simulink model "TosAeb"  (R2021a)
settings: Solver=FixedStepDiscrete  StartTime=0.0  StopTime=inf  FixedStep=0.01  RelTol=1e-3
undefined symbols (not defined in the file or in its folder): ACCModSts, AccSts1, AEB_ObjectMovingOrientation, AEB_ObjectMovingState, CN_BTCActive, CN_CurrentGearR, CN_CurrentGearR_DHT, CN_PTCActive, CN_VDCActive, CN_VSC_EspOff, CN_VSC_FCW_AEB_Error, CN_VSC_FCW_AEB_NoDispay, CN_VSC_FCW_AEB_PerfmDgdtn, CN_VSC_FCW_AEB_TempUnAvlbl, CN_VSC_VehStandstill, CNaccelAvoidMax, CNaccelAvoidMin, CNbrakePedalPress, CNcomfortStrLeftAccelMax, CNcomfortStrLeftAccelMin, CNcomfortStrRightAccelMax, CNcomfortStrRightAccelMin, CNDrvDoorOpen, CNDrvModeEco, CNDrvModeNorm, CNDrvModeOther, CNDrvModeSport, CNDrvSBRreminder, CNNull, CNobjInvalidID, CNPassDoorOpen, CNPassSBRreminder, CNPEBSAccelNegInf, CNPEBSAccelNull, CNPEBSTimeInf, CNPEBSTimeNull, CNSTOPMAX, CNSTOPNULL, CNsubjWidth, CNSwtOFF, CNSwtON, CNTTCMAX, CNTTCNULL, CNVehConfigAEBCycNone, CNVehConfigAEBNone, CNVehConfigAEBPedNone, CNVehConfigFCWNone, CNVehConfigJANone, CollisionSeverity, FALSE, FCWSnvty, JACycObjCalcInfoDefaultParams, JAPedObjCalcInfoDefaultParams, JAVehObjCalcInfoDefaultParams, latCycObjCalcInfoDefaultParams, latPedObjCalcInfoDefaultParams, longObjCalcInfoDefaultParams, ModSts, movingStatus, ObjectType ... (+1112)
libraries: simulink x3031, AEBSFunctionLib x50, Ta_Library x33, AEBSStateMachineLib x23, Mylibrary x12, simulink_extras x7, ObjSelect_lib x3, dspstat3 x2
(part 43 of 102)

== system TosAeb/FcwAebJa_Step_sys/ALG_AEBSystem/ALG_AEBController/AEB_FuncCondCalcApp/PedFunctionCondCalc/pedABASuppAborCond/pedABASuppCondCalc/pedABAObjRelActiveCondCalc ==
  ObjectID : Inport
  vxAbsObj : Inport  Port=2
  ObjFusQuality : Inport  Port=3
  dy : Inport  Port=4
  vy : Inport  Port=5
  Abs : Abs
  Compare To Constant : simulink/Logic and Bit Operations/Compare To Constant  relop=>  const=CNNull  OutDataTypeStr=boolean
  Compare To Constant1 : simulink/Logic and Bit Operations/Compare To Constant  relop=>  const=P_AEB_Func_pedABAVxAbsObjSuppThres  OutDataTypeStr=boolean
  Compare To Constant11 : simulink/Logic and Bit Operations/Compare To Constant  relop===  const=FALSE  OutDataTypeStr=boolean
  Compare To Constant2 : simulink/Logic and Bit Operations/Compare To Constant  relop=>  const=CNNull  OutDataTypeStr=boolean
  Compare To Constant3 : simulink/Logic and Bit Operations/Compare To Constant  relop=<  const=CNNull  OutDataTypeStr=boolean
  Compare To Constant4 : simulink/Logic and Bit Operations/Compare To Constant  relop===  const=CNobjInvalidID  OutDataTypeStr=boolean
  Compare To Constant5 : simulink/Logic and Bit Operations/Compare To Constant  relop=<  const=CNNull  OutDataTypeStr=boolean
  Logical Operator : Logic  Operator=OR  Inputs=3  AllPortsSameDT=off  OutDataTypeStr=boolean
  Logical Operator1 : Logic  AllPortsSameDT=off  OutDataTypeStr=boolean
  Logical Operator2 : Logic  AllPortsSameDT=off  OutDataTypeStr=boolean
  Logical Operator3 : Logic  Operator=OR  AllPortsSameDT=off  OutDataTypeStr=boolean
  Terminator : Terminator
  pedABAObjRelSuppCondFulfilled : Outport
  wires:
  ObjFusQuality/1 -> Compare To Constant11/1
  vy/1 -> Compare To Constant2/1, Compare To Constant5/1
  Compare To Constant2/1 -> Logical Operator1/2
  dy/1 -> Compare To Constant/1, Compare To Constant3/1
  Logical Operator2/1 -> Logical Operator3/2
  Compare To Constant/1 -> Logical Operator1/1
  Compare To Constant3/1 -> Logical Operator2/1
  Logical Operator1/1 -> Logical Operator3/1
  Compare To Constant5/1 -> Logical Operator2/2
  Compare To Constant11/1 -> Logical Operator/3  "ObjFusQuality"
  Logical Operator/1 -> pedABAObjRelSuppCondFulfilled/1
  Compare To Constant4/1 -> Logical Operator/1
  Compare To Constant1/1 -> Logical Operator/2
  vxAbsObj/1 -> Abs/1
  Abs/1 -> Compare To Constant1/1
  ObjectID/1 -> Compare To Constant4/1
  Logical Operator3/1 -> Terminator/1

== system TosAeb/FcwAebJa_Step_sys/ALG_AEBSystem/ALG_AEBController/AEB_FuncCondCalcApp/PedFunctionCondCalc/pedABASuppAborCond/pedABASuppCondCalc/pedABASituationRelActiveCondCalc ==
  latCollisionSeverity : Inport
  subjCurvTraj : Inport  Port=2
  subjLatAccel : Inport  Port=3
  ObjectTypeCameraConfirmed : Inport  Port=4
  vy : Inport  Port=5
  dxFrontBumper : Inport  Port=6
  colliisonRiskProb : Inport  Port=7
  FcwHMISetting : Inport  Port=8
  subjVel : Inport  Port=9
  LongDistCond4PreWarningABA : Subsystem
  pedABASituationRelSuppCondCalc : Subsystem
  vyCorrCalc : Subsystem
  pedABASituationRelSuppCondFulfilled : Outport
  wires:
  LongDistCond4PreWarningABA/1(longDistCond4ABA) -> pedABASituationRelSuppCondCalc/7(objLongDistCond4ABA)
  vyCorrCalc/1(objLatVyCond4ABA) -> pedABASituationRelSuppCondCalc/6(pedLatVyCond4ABA)
  pedABASituationRelSuppCondCalc/1(pedABASituationRelSuppCondFulfilled) -> pedABASituationRelSuppCondFulfilled/1
  colliisonRiskProb/1 -> pedABASituationRelSuppCondCalc/4(colliisonRiskProb)
  subjLatAccel/1 -> pedABASituationRelSuppCondCalc/3(subjLatAccel)
  subjCurvTraj/1 -> pedABASituationRelSuppCondCalc/2(subjCurvTraj)
  latCollisionSeverity/1 -> pedABASituationRelSuppCondCalc/1(latCollisionSeverity)
  subjVel/1 -> LongDistCond4PreWarningABA/2(subjVel)
  FcwHMISetting/1 -> LongDistCond4PreWarningABA/1(FcwHMISetting)
  dxFrontBumper/1 -> LongDistCond4PreWarningABA/3(dxFrontBumper)  "dxFrontBumper"
  ObjectTypeCameraConfirmed/1 -> pedABASituationRelSuppCondCalc/5(ObjectTypeCameraConfirmed), vyCorrCalc/1(ObjectTypeCameraConfirmed)
  vy/1 -> vyCorrCalc/2(vy)

== system TosAeb/FcwAebJa_Step_sys/ALG_AEBSystem/ALG_AEBController/AEB_FuncCondCalcApp/PedFunctionCondCalc/pedABASuppAborCond/pedABASuppCondCalc/pedABASuppCondFinalJudge ==
  pedABAObjRelSuppCondFulfilled : Inport
  pedABAVehRelSuppCondFulfilled : Inport  Port=2
  pedABASituationRelSuppCondFulfilled : Inport  Port=3
  Compare To Constant : simulink/Logic and Bit Operations/Compare To Constant  relop===  const=TRUE  OutDataTypeStr=boolean
  Compare To Constant1 : simulink/Logic and Bit Operations/Compare To Constant  relop===  const=TRUE  OutDataTypeStr=boolean
  Compare To Constant2 : simulink/Logic and Bit Operations/Compare To Constant  relop===  const=TRUE  OutDataTypeStr=boolean
  Logical Operator : Logic  Operator=OR  Inputs=3  AllPortsSameDT=off  OutDataTypeStr=boolean
  pedABASuppCondFulfilled : Outport
  wires:
  Logical Operator/1 -> pedABASuppCondFulfilled/1
  pedABASituationRelSuppCondFulfilled/1 -> Compare To Constant2/1
  pedABAObjRelSuppCondFulfilled/1 -> Compare To Constant/1
  pedABAVehRelSuppCondFulfilled/1 -> Compare To Constant1/1
  Compare To Constant/1 -> Logical Operator/1
  Compare To Constant1/1 -> Logical Operator/2
  Compare To Constant2/1 -> Logical Operator/3

== system TosAeb/FcwAebJa_Step_sys/ALG_AEBSystem/ALG_AEBController/AEB_FuncCondCalcApp/PedFunctionCondCalc/pedABASuppAborCond/pedABASuppCondCalc/pedABAVehRelActiveCondCalc ==
  subjVel : Inport
  SteerWheelAngle : Inport  Port=2
  SteerWheelSpd : Inport  Port=3
  AccrPedlPosnDiagc : Inport  Port=4
  vehStateCalcPedABASuppFlg : Inport  Port=5
  Abs : Abs
  Abs1 : Abs
  AccReleventSuppCondDelayAbortion : AEBSFunctionLib/StateDelayAbortion  SystemSampleTime=-1  VariantActivationTime=update diagram  Latency=0  AutoFrameSizeCalculation=off
  Compare To Constant : simulink/Logic and Bit Operations/Compare To Constant  relop=>  const=P_AEB_Func_pedABAStrWheelAngleSupThres  OutDataTypeStr=boolean
  Compare To Constant1 : simulink/Logic and Bit Operations/Compare To Constant  relop=>  const=P_AEB_Func_pedABAGasPedalSupThres  OutDataTypeStr=boolean
  Compare To Constant2 : simulink/Logic and Bit Operations/Compare To Constant  relop=>  const=P_AEB_Func_pedABAStrWheelAngleSpdSupThres  OutDataTypeStr=boolean
  Compare To Constant7 : simulink/Logic and Bit Operations/Compare To Constant  relop===  const=TRUE  OutDataTypeStr=boolean
  Compare To Constant8 : simulink/Logic and Bit Operations/Compare To Constant  relop=>  const=P_AEB_Func_pedABASubjVelMax  OutDataTypeStr=boolean
  Compare To Constant9 : simulink/Logic and Bit Operations/Compare To Constant  relop=<  const=P_AEB_Func_pedABASubjVelMin  OutDataTypeStr=boolean
  Constant : Constant  Value=P_AEB_Func_pedABAStrCondAborDelayTime
  Constant1 : Constant  Value=0.02
  Constant2 : Constant  Value=P_AEB_Func_pedABAAccrCondAborDelayTime
  Constant3 : Constant  Value=0.02
  Logical Operator : Logic  Operator=OR  AllPortsSameDT=off  OutDataTypeStr=boolean
  Logical Operator4 : Logic  Operator=OR  AllPortsSameDT=off  OutDataTypeStr=boolean
  Logical Operator5 : Logic  Operator=OR  Inputs=4  AllPortsSameDT=off  OutDataTypeStr=boolean
  StrReleventSuppCondDelayAbortion : AEBSFunctionLib/StateDelayAbortion  SystemSampleTime=-1  VariantActivationTime=update diagram  Latency=0  AutoFrameSizeCalculation=off
  pedABAVehRelSuppCondFulfilled : Outport
  wires:
  Compare To Constant1/1 -> AccReleventSuppCondDelayAbortion/1
  Constant2/1 -> AccReleventSuppCondDelayAbortion/2
  Constant3/1 -> AccReleventSuppCondDelayAbortion/3
  AccrPedlPosnDiagc/1 -> Compare To Constant1/1
  Logical Operator4/1 -> StrReleventSuppCondDelayAbortion/1
  Constant1/1 -> StrReleventSuppCondDelayAbortion/3
  Constant/1 -> StrReleventSuppCondDelayAbortion/2
  Logical Operator5/1 -> pedABAVehRelSuppCondFulfilled/1  "pedPrefillVehRelSuppCondFulfilled"
  subjVel/1 -> Compare To Constant9/1, Compare To Constant8/1
  Logical Operator/1 -> Logical Operator5/1  "subjVelReleventSuppCondFulfilled"
  Compare To Constant7/1 -> Logical Operator5/4  "vehStateCalcPedPrefillSuppCondFulfilled"
  Compare To Constant9/1 -> Logical Operator/2
  StrReleventSuppCondDelayAbortion/1 -> Logical Operator5/2  "strReleventSuppCondFulfilled"
  SteerWheelAngle/1 -> Abs/1
  Abs/1 -> Compare To Constant/1
  Compare To Constant/1 -> Logical Operator4/1
  SteerWheelSpd/1 -> Abs1/1
  Compare To Constant2/1 -> Logical Operator4/2
  Compare To Constant8/1 -> Logical Operator/1
  vehStateCalcPedABASuppFlg/1 -> Compare To Constant7/1
  Abs1/1 -> Compare To Constant2/1
  AccReleventSuppCondDelayAbortion/1 -> Logical Operator5/3  "accReleventSuppCondFulfilled"

== system TosAeb/FcwAebJa_Step_sys/ALG_AEBSystem/ALG_AEBController/AEB_FuncCondCalcApp/PedFunctionCondCalc/pedAEBfullSuppAborCond/pedAEBfullAborCondNoStandstillCalc/pedAEBfullAborCondFinalJudge ==
  pedAEBfullObjRelAborCondFulfilled : Inport
  pedAEBfullVehRelAborCondFulfilled : Inport  Port=2
  pedAEBfullSituationRelAborCondFulfilled : Inport  Port=3
  Compare To Constant1 : simulink/Logic and Bit Operations/Compare To Constant  relop===  const=TRUE  OutDataTypeStr=boolean
  Compare To Constant2 : simulink/Logic and Bit Operations/Compare To Constant  relop===  const=TRUE  OutDataTypeStr=boolean
  Compare To Constant3 : simulink/Logic and Bit Operations/Compare To Constant  relop===  const=TRUE  OutDataTypeStr=boolean
  Logical Operator : Logic  Operator=OR  Inputs=3  AllPortsSameDT=off  OutDataTypeStr=boolean
  pedAEBfullAborCondNoStandstillFulfilled : Outport
  wires:
  Compare To Constant2/1 -> Logical Operator/3
  Compare To Constant1/1 -> Logical Operator/2
  pedAEBfullObjRelAborCondFulfilled/1 -> Compare To Constant3/1
  Logical Operator/1 -> pedAEBfullAborCondNoStandstillFulfilled/1  "pedAEBfullAborCondNoStandstillFulfilled"
  pedAEBfullSituationRelAborCondFulfilled/1 -> Compare To Constant2/1
  pedAEBfullVehRelAborCondFulfilled/1 -> Compare To Constant1/1
  Compare To Constant3/1 -> Logical Operator/1

== system TosAeb/FcwAebJa_Step_sys/ALG_AEBSystem/ALG_AEBController/AEB_FuncCondCalcApp/PedFunctionCondCalc/pedAEBfullSuppAborCond/pedAEBfullAborCondNoStandstillCalc/pedAEBfullObjRelActiveCondCalc ==
  ObjectID : Inport
  objIsFusionTarget : Inport  Port=2
  latCollsionRiskProb : Inport  Port=3
  Chart : Stateflow chart  TreatAsAtomicUnit=on
  Compare To Constant : simulink/Logic and Bit Operations/Compare To Constant  relop===  const=TRUE  OutDataTypeStr=boolean
  Constant : Constant  Value=P_AEB_Func_pedAEBfulColliisonRiskProbAborThresObjID
  Unit Delay : UnitDelay  SampleTime=-1  HasFrameUpgradeWarning=on
  pedAEBfullObjRelAborCondFulfilled : Outport
  wires:
  Constant/1 -> Chart/5(P_pedAEBfulColliisonRiskProbAborThresObjID)
  Compare To Constant/1 -> Chart/3(objIsFusionTarget)
  objIsFusionTarget/1 -> Compare To Constant/1
  ObjectID/1 -> Chart/1(targetObjID), Unit Delay/1
  Chart/1(pedAEBfullObjRelAborCondFulfilled) -> pedAEBfullObjRelAborCondFulfilled/1
  Unit Delay/1 -> Chart/2(targetObjIDObjCalcK1)
  latCollsionRiskProb/1 -> Chart/4(objLatCollisionProb)

== system TosAeb/FcwAebJa_Step_sys/ALG_AEBSystem/ALG_AEBController/AEB_FuncCondCalcApp/PedFunctionCondCalc/pedAEBfullSuppAborCond/pedAEBfullAborCondNoStandstillCalc/pedAEBfullSituationRelAborCondCalc ==
  pedVehVelReduced : Inport
  pedCollisionSeverity : Inport  Port=2
  objLatCollsionRiskProb : Inport  Port=3
  dy : Inport  Port=4
  vy : Inport  Port=5
  dx : Inport  Port=6
  subjYawRate : Inport  Port=7
  Abs : Abs
  Abs1 : Abs
  Add : Sum
  Compare To Constant : simulink/Logic and Bit Operations/Compare To Constant  relop===  const=CollisionSeverity.None  OutDataTypeStr=boolean
  Compare To Constant1 : simulink/Logic and Bit Operations/Compare To Constant  relop=>  const=P_AEB_Func_pedAEBfullVehVelReduceAborThres  OutDataTypeStr=boolean
  Compare To Constant2 : simulink/Logic and Bit Operations/Compare To Constant  relop=>  const=P_AEB_Func_pedAEBfullAbsDyAborThres  OutDataTypeStr=boolean
  Compare To Constant3 : simulink/Logic and Bit Operations/Compare To Constant  relop=<  const=P_AEB_Func_pedAEBfullColliisonRiskProbAborThres  OutDataTypeStr=boolean
  Compare To Constant4 : simulink/Logic and Bit Operations/Compare To Constant  relop=<  const=P_AEB_Func_pedAEBfullAbsVyAborThres  OutDataTypeStr=boolean
  Logical Operator : Logic  AllPortsSameDT=off  OutDataTypeStr=boolean
  Logical Operator1 : Logic  Operator=OR  Inputs=4  AllPortsSameDT=off  OutDataTypeStr=boolean
  Product : Product
  pedAEBfullSituationRelAborCondFulfilled : Outport
  wires:
  Compare To Constant1/1 -> Logical Operator1/1
  Compare To Constant/1 -> Logical Operator1/2
  Add/1 -> Abs1/1  "vyCorr"
  dx/1 -> Product/1
  subjYawRate/1 -> Product/2
  vy/1 -> Add/1
  Product/1 -> Add/2
  Logical Operator1/1 -> pedAEBfullSituationRelAborCondFulfilled/1
  Compare To Constant3/1 -> Logical Operator1/3
  pedCollisionSeverity/1 -> Compare To Constant/1
  objLatCollsionRiskProb/1 -> Compare To Constant3/1
  pedVehVelReduced/1 -> Compare To Constant1/1
  dy/1 -> Abs/1
  Abs/1 -> Compare To Constant2/1
  Abs1/1 -> Compare To Constant4/1
  Compare To Constant2/1 -> Logical Operator/1
  Compare To Constant4/1 -> Logical Operator/2
  Logical Operator/1 -> Logical Operator1/4

== system TosAeb/FcwAebJa_Step_sys/ALG_AEBSystem/ALG_AEBController/AEB_FuncCondCalcApp/PedFunctionCondCalc/pedAEBfullSuppAborCond/pedAEBfullAborCondNoStandstillCalc/pedAEBfullVehRelAborCondCalc ==
  note: 取消自车速度判断条件
  SteerWheelAngle : Inport
  SteerWheelSpd : Inport  Port=2
  vehStateCalcAEBfullAborFlg : Inport  Port=3
  pedAEBfullWorkTime : Inport  Port=4
  vehStateCalcStandstill : Inport  Port=5
  Abs : Abs
  Abs1 : Abs
  Compare To Constant : simulink/Logic and Bit Operations/Compare To Constant  relop=>  const=P_AEB_Func_pedAEBfullStrWheelAngleAborThres  OutDataTypeStr=boolean
  Compare To Constant1 : simulink/Logic and Bit Operations/Compare To Constant  relop=>  const=P_AEB_Func_pedAEBfullStrWheelAngleSpdAborThres  OutDataTypeStr=boolean
  Compare To Constant2 : simulink/Logic and Bit Operations/Compare To Constant  relop=>  const=P_AEB_Func_pedAEBfullActivationTimeAborThres  OutDataTypeStr=boolean
  Compare To Constant3 : simulink/Logic and Bit Operations/Compare To Constant  relop===  const=TRUE  OutDataTypeStr=boolean
  Compare To Constant4 : simulink/Logic and Bit Operations/Compare To Constant  relop===  const=FALSE  OutDataTypeStr=boolean
  Logical Operator : Logic  Operator=OR  AllPortsSameDT=off  OutDataTypeStr=boolean
  Logical Operator2 : Logic  Operator=OR  Inputs=3  AllPortsSameDT=off  OutDataTypeStr=boolean
  Logical Operator4 : Logic  AllPortsSameDT=off  OutDataTypeStr=boolean
  pedAEBfullVehRelAborCondFulfilled : Outport
  wires:
  Compare To Constant3/1 -> Logical Operator2/2  "vehStateCalcAEBfullAborFlg"
  Logical Operator4/1 -> Logical Operator2/3  "pedPrefillWorkTimeRelCondFulfilled"
  Abs/1 -> Compare To Constant/1
  SteerWheelSpd/1 -> Abs1/1
  Abs1/1 -> Compare To Constant1/1
  Compare To Constant1/1 -> Logical Operator/2
  SteerWheelAngle/1 -> Abs/1
  pedAEBfullWorkTime/1 -> Compare To Constant2/1
  vehStateCalcAEBfullAborFlg/1 -> Compare To Constant3/1
  Compare To Constant/1 -> Logical Operator/1
  Logical Operator/1 -> Logical Operator2/1  "strReleventAborCondFulfilled"
  Logical Operator2/1 -> pedAEBfullVehRelAborCondFulfilled/1  "pedPrefillVehRelAborCondFulfilled"
  vehStateCalcStandstill/1 -> Compare To Constant4/1
  Compare To Constant4/1 -> Logical Operator4/1
  Compare To Constant2/1 -> Logical Operator4/2

== system TosAeb/FcwAebJa_Step_sys/ALG_AEBSystem/ALG_AEBController/AEB_FuncCondCalcApp/PedFunctionCondCalc/pedAEBfullSuppAborCond/pedAEBfullAborCondStandstillCalc/pedAEBfullVehRelAborCondCalc ==
  note: 取消速度限制
  subjVel : Inport
  SteerWheelAngle : Inport  Port=2
  SteerWheelSpd : Inport  Port=3
  vehStateCalcAEBfullAborFlg : Inport  Port=4
  pedAEBfullWorkTime : Inport  Port=5
  vehStateCalcStandstill : Inport  Port=6
  Abs : Abs
  Abs1 : Abs
  Compare To Constant : simulink/Logic and Bit Operations/Compare To Constant  relop=>  const=P_AEB_Func_pedAEBfullStrWheelAngleAborThresStandstill  OutDataTypeStr=boolean
  Compare To Constant1 : simulink/Logic and Bit Operations/Compare To Constant  relop=>  const=P_AEB_Func_pedAEBfullStrWheelAngleSpdAborThresStandstill  OutDataTypeStr=boolean
  Compare To Constant2 : simulink/Logic and Bit Operations/Compare To Constant  relop=>  const=P_AEB_Func_pedAEBfullActivationTimeAborThresStandstill  OutDataTypeStr=boolean
  Compare To Constant3 : simulink/Logic and Bit Operations/Compare To Constant  relop===  const=TRUE  OutDataTypeStr=boolean
  Compare To Constant4 : simulink/Logic and Bit Operations/Compare To Constant  relop===  const=TRUE  OutDataTypeStr=boolean
  Compare To Constant7 : simulink/Logic and Bit Operations/Compare To Constant  relop=<  const=P_AEB_Func_pedAEBfullSubjVelAborThresStandstill  OutDataTypeStr=boolean
  Logical Operator : Logic  Operator=OR  AllPortsSameDT=off  OutDataTypeStr=boolean
  Logical Operator2 : Logic  Operator=OR  Inputs=3  AllPortsSameDT=off  OutDataTypeStr=boolean
  Logical Operator4 : Logic  Inputs=3  AllPortsSameDT=off  OutDataTypeStr=boolean
  pedAEBfullVehRelAborCondFulfilled : Outport
  wires:
  Logical Operator4/1 -> Logical Operator2/3  "pedPrefillWorkTimeRelCondFulfilled"
  vehStateCalcStandstill/1 -> Compare To Constant4/1
  subjVel/1 -> Compare To Constant7/1
  Logical Operator2/1 -> pedAEBfullVehRelAborCondFulfilled/1  "pedPrefillVehRelAborCondFulfilled"
  Logical Operator/1 -> Logical Operator2/1  "strReleventAborCondFulfilled"
  Compare To Constant/1 -> Logical Operator/1
  vehStateCalcAEBfullAborFlg/1 -> Compare To Constant3/1
  pedAEBfullWorkTime/1 -> Compare To Constant2/1
  SteerWheelAngle/1 -> Abs/1
  Compare To Constant1/1 -> Logical Operator/2
  Abs1/1 -> Compare To Constant1/1
  SteerWheelSpd/1 -> Abs1/1
  Abs/1 -> Compare To Constant/1
  Compare To Constant3/1 -> Logical Operator2/2  "vehStateCalcAEBfullAborFlg"
  Compare To Constant4/1 -> Logical Operator4/2
  Compare To Constant7/1 -> Logical Operator4/1
  Compare To Constant2/1 -> Logical Operator4/3

== system TosAeb/FcwAebJa_Step_sys/ALG_AEBSystem/ALG_AEBController/AEB_FuncCondCalcApp/PedFunctionCondCalc/pedAEBfullSuppAborCond/pedAEBfullSuppCondCalc/pedAEBfullObjRelSuppCondCalc ==
  ObjectID : Inport
  vxAbsObj : Inport  Port=2
  ObjFusQuality : Inport  Port=3
  dy : Inport  Port=4
  vy : Inport  Port=5
  Abs : Abs
  Compare To Constant : simulink/Logic and Bit Operations/Compare To Constant  relop=>  const=CNNull  OutDataTypeStr=boolean
  Compare To Constant1 : simulink/Logic and Bit Operations/Compare To Constant  relop=>  const=P_AEB_Func_pedAEBfullVxAbsObjSuppThres  OutDataTypeStr=boolean
  Compare To Constant11 : simulink/Logic and Bit Operations/Compare To Constant  relop===  const=FALSE  OutDataTypeStr=boolean
  Compare To Constant2 : simulink/Logic and Bit Operations/Compare To Constant  relop=>  const=CNNull  OutDataTypeStr=boolean
  Compare To Constant3 : simulink/Logic and Bit Operations/Compare To Constant  relop=<  const=CNNull  OutDataTypeStr=boolean
  Compare To Constant4 : simulink/Logic and Bit Operations/Compare To Constant  relop===  const=CNobjInvalidID  OutDataTypeStr=boolean
  Compare To Constant5 : simulink/Logic and Bit Operations/Compare To Constant  relop=<  const=CNNull  OutDataTypeStr=boolean
  Logical Operator : Logic  Operator=OR  Inputs=3  AllPortsSameDT=off  OutDataTypeStr=boolean
  Logical Operator1 : Logic  AllPortsSameDT=off  OutDataTypeStr=boolean
  Logical Operator2 : Logic  AllPortsSameDT=off  OutDataTypeStr=boolean
  Logical Operator3 : Logic  Operator=OR  AllPortsSameDT=off  OutDataTypeStr=boolean
  Terminator : Terminator
  pedAEBfullObjRelSuppCondFulfilled : Outport
  wires:
  ObjFusQuality/1 -> Compare To Constant11/1
  vy/1 -> Compare To Constant2/1, Compare To Constant5/1
  Compare To Constant2/1 -> Logical Operator1/2
  dy/1 -> Compare To Constant/1, Compare To Constant3/1
  Logical Operator2/1 -> Logical Operator3/2
  Compare To Constant/1 -> Logical Operator1/1
  Compare To Constant3/1 -> Logical Operator2/1
  Logical Operator1/1 -> Logical Operator3/1
  Compare To Constant5/1 -> Logical Operator2/2
  Compare To Constant11/1 -> Logical Operator/3  "ObjFusQuality"
  vxAbsObj/1 -> Abs/1
  Compare To Constant1/1 -> Logical Operator/2
  Abs/1 -> Compare To Constant1/1
  Compare To Constant4/1 -> Logical Operator/1
  Logical Operator/1 -> pedAEBfullObjRelSuppCondFulfilled/1
  ObjectID/1 -> Compare To Constant4/1
  Logical Operator3/1 -> Terminator/1

== system TosAeb/FcwAebJa_Step_sys/ALG_AEBSystem/ALG_AEBController/AEB_FuncCondCalcApp/PedFunctionCondCalc/pedAEBfullSuppAborCond/pedAEBfullSuppCondCalc/pedAEBfullSituationRelSuppCondCalc ==
  latCollisionSeverity : Inport
  subjCurvTraj : Inport  Port=2
  subjLatAccel : Inport  Port=3
  pedCollisionProbFlg : Inport  Port=4
  pedCollisionRisk : Inport  Port=5
  dy : Inport  Port=6
  FcwHMISetting : Inport  Port=7
  subjVel : Inport  Port=8
  dxFrontBumper : Inport  Port=9
  ObjectTypeCameraConfirmed : Inport  Port=10
  distSecure : Inport  Port=11
  distBraking : Inport  Port=12
  vy : Inport  Port=13
  dy_ConfirmCalc : Subsystem  Commented=on
  latDistCondCalc : Subsystem
  longDistCond4fullBrkCalc : Subsystem
  objLatVyCond : Subsystem
  pedAEBfullSituationRelSuppCond : Subsystem
  vy_ConfirmCalc : Subsystem  Commented=on
  pedAEBfullSituationRelSuppCondFulfilled : Outport
  wires:
  [dy] -> dy_ConfirmCalc/3(dy)
  [subjVel] -> dy_ConfirmCalc/1(subjVel)
  [dy] -> vy_ConfirmCalc/2(dy)
  dxFrontBumper/1 -> longDistCond4fullBrkCalc/1(dxFrontBumper)
  [subjVel] -> longDistCond4fullBrkCalc/4(subjVel)
  distBraking/1 -> longDistCond4fullBrkCalc/3(distBraking)
  distSecure/1 -> longDistCond4fullBrkCalc/2(distSecure)
  longDistCond4fullBrkCalc/2(longDistMinCond4Brake) -> pedAEBfullSituationRelSuppCond/10(distMinCond4FullBrake)
  longDistCond4fullBrkCalc/1(longDistCond4fullBrk) -> pedAEBfullSituationRelSuppCond/9(objLongDistCond4FullBrake)
  latDistCondCalc/1(latDistCond) -> pedAEBfullSituationRelSuppCond/8(latDistCond)
  objLatVyCond/1(objLatVyCond4FullBrake) -> pedAEBfullSituationRelSuppCond/7(objLatVyCond4FullBrake)
  ObjectTypeCameraConfirmed/1 -> objLatVyCond/1(ObjectTypeCameraConfirmed), pedAEBfullSituationRelSuppCond/6(ObjectTypeCameraConfirmed)
  pedAEBfullSituationRelSuppCond/1(pedAEBfullSituationRelSuppCondFulfilled) -> pedAEBfullSituationRelSuppCondFulfilled/1
  pedCollisionRisk/1 -> pedAEBfullSituationRelSuppCond/5(pedCollisionRisk)
  pedCollisionProbFlg/1 -> pedAEBfullSituationRelSuppCond/4(pedCollisionProbFlg)
  subjLatAccel/1 -> pedAEBfullSituationRelSuppCond/3(subjLatAccel)
  subjCurvTraj/1 -> pedAEBfullSituationRelSuppCond/2(subjCurvTraj)
  latCollisionSeverity/1 -> pedAEBfullSituationRelSuppCond/1(latCollisionSeverity)
  dy/1 -> objLatVyCond/3(dy), latDistCondCalc/1(dy), [dy]
  FcwHMISetting/1 -> latDistCondCalc/2(FcwHMISetting)
  subjVel/1 -> latDistCondCalc/3(subjVel), [subjVel]
  vy/1 -> latDistCondCalc/4(vy), vy_ConfirmCalc/1(vy), objLatVyCond/2(vy)

== system TosAeb/FcwAebJa_Step_sys/ALG_AEBSystem/ALG_AEBController/AEB_FuncCondCalcApp/PedFunctionCondCalc/pedAEBfullSuppAborCond/pedAEBfullSuppCondCalc/pedAEBfullSuppCondFinalJudge ==
  pedAEBfullObjRelSuppCondFulfilled : Inport
  pedAEBfullVehRelSuppCondFulfilled : Inport  Port=2
  pedAEBfullSituationRelSuppCondFulfilled : Inport  Port=3
  Compare To Constant : simulink/Logic and Bit Operations/Compare To Constant  relop===  const=TRUE  OutDataTypeStr=boolean
  Compare To Constant1 : simulink/Logic and Bit Operations/Compare To Constant  relop===  const=TRUE  OutDataTypeStr=boolean
  Compare To Constant2 : simulink/Logic and Bit Operations/Compare To Constant  relop===  const=TRUE  OutDataTypeStr=boolean
  Logical Operator : Logic  Operator=OR  Inputs=3  AllPortsSameDT=off  OutDataTypeStr=boolean
  pedAEBfullSuppCondFulfilled : Outport
  wires:
  Logical Operator/1 -> pedAEBfullSuppCondFulfilled/1
  pedAEBfullSituationRelSuppCondFulfilled/1 -> Compare To Constant2/1
  pedAEBfullObjRelSuppCondFulfilled/1 -> Compare To Constant/1
  pedAEBfullVehRelSuppCondFulfilled/1 -> Compare To Constant1/1
  Compare To Constant/1 -> Logical Operator/1
  Compare To Constant1/1 -> Logical Operator/2
  Compare To Constant2/1 -> Logical Operator/3

== system TosAeb/FcwAebJa_Step_sys/ALG_AEBSystem/ALG_AEBController/AEB_FuncCondCalcApp/PedFunctionCondCalc/pedAEBfullSuppAborCond/pedAEBfullSuppCondCalc/pedAEBfullVehRelSuppCondCalc ==
  subjVel : Inport
  VehConfigIbooster : Inport  Port=2
  ESP_MasterCylindBrakePressValid : Inport  Port=3
  ESP_MasterCylindBrakePress : Inport  Port=4
  SteerWheelAngle : Inport  Port=5
  SteerWheelSpd : Inport  Port=6
  AccrPedlPosnDiagc : Inport  Port=7
  vehStateCalcPedAEBfullSuppFlg : Inport  Port=8
  Abs : Abs
  Abs1 : Abs
  AccReleventSuppCondDelayAbortion : AEBSFunctionLib/StateDelayAbortion  SystemSampleTime=-1  VariantActivationTime=update diagram  Latency=0  AutoFrameSizeCalculation=off
  Compare To Constant : simulink/Logic and Bit Operations/Compare To Constant  relop=>  const=P_AEB_Func_pedAEBfullStrWheelAngleSupThres  OutDataTypeStr=boolean
  Compare To Constant1 : simulink/Logic and Bit Operations/Compare To Constant  relop=>  const=P_AEB_Func_pedAEBfullGasPedalSupThres  OutDataTypeStr=boolean
  Compare To Constant2 : simulink/Logic and Bit Operations/Compare To Constant  relop=>  const=P_AEB_Func_pedAEBfullStrWheelAngleSpdSupThres  OutDataTypeStr=boolean
  Compare To Constant7 : simulink/Logic and Bit Operations/Compare To Constant  relop===  const=TRUE  OutDataTypeStr=boolean
  Compare To Constant8 : simulink/Logic and Bit Operations/Compare To Constant  relop=>  const=P_AEB_Func_pedAEBfullSubjVelMax  OutDataTypeStr=boolean
  Compare To Constant9 : simulink/Logic and Bit Operations/Compare To Constant  relop=<  const=P_AEB_Func_pedAEBfullSubjVelMin  OutDataTypeStr=boolean
  Constant : Constant  Value=P_AEB_Func_pedAEBfullStrCondAborDelayTime
  Constant1 : Constant  Value=0.02
  Constant2 : Constant  Value=P_AEB_Func_pedAEBfullStrCondAborDelayTime
  Constant3 : Constant  Value=0.02
  Logical Operator : Logic  Operator=OR  AllPortsSameDT=off  OutDataTypeStr=boolean
  Logical Operator4 : Logic  Operator=OR  AllPortsSameDT=off  OutDataTypeStr=boolean
  Logical Operator5 : Logic  Operator=OR  Inputs=5  AllPortsSameDT=off  OutDataTypeStr=boolean
  StrReleventSuppCondDelayAbortion : AEBSFunctionLib/StateDelayAbortion  SystemSampleTime=-1  VariantActivationTime=update diagram  Latency=0  AutoFrameSizeCalculation=off
  brakeReleventSuppCond : Subsystem
  pedAEBfullVehRelSuppCondFulfilled : Outport
  wires:
  VehConfigIbooster/1 -> brakeReleventSuppCond/1(VehConfigIbooster)
  ESP_MasterCylindBrakePress/1 -> brakeReleventSuppCond/3(ESP_MasterCylindBrakePress)
  ESP_MasterCylindBrakePressValid/1 -> brakeReleventSuppCond/2(ESP_MasterCylindBrakePressValid)
  Compare To Constant1/1 -> AccReleventSuppCondDelayAbortion/1
  Constant2/1 -> AccReleventSuppCondDelayAbortion/2
  Constant3/1 -> AccReleventSuppCondDelayAbortion/3
  AccrPedlPosnDiagc/1 -> Compare To Constant1/1
  Logical Operator4/1 -> StrReleventSuppCondDelayAbortion/1
  Constant1/1 -> StrReleventSuppCondDelayAbortion/3
  Constant/1 -> StrReleventSuppCondDelayAbortion/2
  Logical Operator5/1 -> pedAEBfullVehRelSuppCondFulfilled/1  "pedAEBfullVehRelSuppCondFulfilled"
  subjVel/1 -> Compare To Constant9/1, Compare To Constant8/1
  Logical Operator/1 -> Logical Operator5/1  "subjVelReleventSuppCondFulfilled"
  Compare To Constant7/1 -> Logical Operator5/5  "vehStateCalcPedPrefillSuppCondFulfilled"
  Compare To Constant9/1 -> Logical Operator/2
  brakeReleventSuppCond/1(brakeReleventSuppCond) -> Logical Operator5/2  "BrkReleventSuppCondFulfilled"
  StrReleventSuppCondDelayAbortion/1 -> Logical Operator5/3  "strReleventSuppCondFulfilled"
  SteerWheelAngle/1 -> Abs/1
  Abs/1 -> Compare To Constant/1
  Compare To Constant/1 -> Logical Operator4/1
  SteerWheelSpd/1 -> Abs1/1
  Compare To Constant2/1 -> Logical Operator4/2
  Compare To Constant8/1 -> Logical Operator/1
  vehStateCalcPedAEBfullSuppFlg/1 -> Compare To Constant7/1
  Abs1/1 -> Compare To Constant2/1
  AccReleventSuppCondDelayAbortion/1 -> Logical Operator5/4  "accReleventSuppCondFulfilled"

== system TosAeb/FcwAebJa_Step_sys/ALG_AEBSystem/ALG_AEBController/AEB_FuncCondCalcApp/PedFunctionCondCalc/pedPreWarningSuppAborCond/pedPreWarningAborCondCalc/pedPreWarningAborCondFinalJudge ==
  pedPreWarningObjRelAborCondFulfilled : Inport
  pedPreWarningVehRelAborCondFulfilled : Inport  Port=2
  pedPreWarningSituationRelAborCondFulfilled : Inport  Port=3
  Compare To Constant1 : simulink/Logic and Bit Operations/Compare To Constant  relop===  const=TRUE  OutDataTypeStr=boolean
  Compare To Constant2 : simulink/Logic and Bit Operations/Compare To Constant  relop===  const=TRUE  OutDataTypeStr=boolean
  Compare To Constant3 : simulink/Logic and Bit Operations/Compare To Constant  relop===  const=TRUE  OutDataTypeStr=boolean
  Logical Operator : Logic  Operator=OR  Inputs=3  AllPortsSameDT=off  OutDataTypeStr=boolean
  pedPreWarningAborCondFulfilled : Outport
  wires:
  Compare To Constant3/1 -> Logical Operator/1
  pedPreWarningVehRelAborCondFulfilled/1 -> Compare To Constant1/1
  pedPreWarningSituationRelAborCondFulfilled/1 -> Compare To Constant2/1
  Logical Operator/1 -> pedPreWarningAborCondFulfilled/1  "vehPreWarningAborCondFulfilled"
  pedPreWarningObjRelAborCondFulfilled/1 -> Compare To Constant3/1
  Compare To Constant1/1 -> Logical Operator/2
  Compare To Constant2/1 -> Logical Operator/3

== system TosAeb/FcwAebJa_Step_sys/ALG_AEBSystem/ALG_AEBController/AEB_FuncCondCalcApp/PedFunctionCondCalc/pedPreWarningSuppAborCond/pedPreWarningAborCondCalc/pedPreWarningObjRelActiveCondCalc ==
  ObjectID : Inport
  pedPreWarningWorkTime : Inport  Port=2
  Compare To Constant : simulink/Logic and Bit Operations/Compare To Constant  relop=>=  const=P_AEB_Func_pedPrewarningMinActivationTimeAborThres  OutDataTypeStr=boolean
  Compare To Constant1 : simulink/Logic and Bit Operations/Compare To Constant  relop=>=  const=P_AEB_Func_pedPrewarningMinActivationTimeAborThresObjID  OutDataTypeStr=boolean
  Compare To Constant4 : simulink/Logic and Bit Operations/Compare To Constant  relop=~=  const=CNobjInvalidID  OutDataTypeStr=boolean
  Logical Operator : Logic  AllPortsSameDT=off  OutDataTypeStr=boolean
  Relational Operator : RelationalOperator  Operator=~=  OutDataTypeStr=boolean  RndMeth=Simplest
  Switch : Switch  Criteria=u2 ~= 0
  Unit Delay : UnitDelay  SampleTime=-1  HasFrameUpgradeWarning=on
  pedPreWarningObjRelAborCondFulfilled : Outport
  wires:
  Compare To Constant4/1 -> Switch/2
  pedPreWarningWorkTime/1 -> Compare To Constant/1, Compare To Constant1/1
  Compare To Constant/1 -> Switch/3
  ObjectID/1 -> Unit Delay/1, Relational Operator/2, Compare To Constant4/1
  Unit Delay/1 -> Relational Operator/1
  Compare To Constant1/1 -> Logical Operator/1
  Relational Operator/1 -> Logical Operator/2
  Switch/1 -> pedPreWarningObjRelAborCondFulfilled/1
  Logical Operator/1 -> Switch/1

== system TosAeb/FcwAebJa_Step_sys/ALG_AEBSystem/ALG_AEBController/AEB_FuncCondCalcApp/PedFunctionCondCalc/pedPreWarningSuppAborCond/pedPreWarningAborCondCalc/pedPreWarningSituationRelAborCondCalc ==
  note: 将true改为false
  latCollisionSeverity : Inport
  pedPreWarningWorkTime : Inport  Port=2
  Compare To Constant : simulink/Logic and Bit Operations/Compare To Constant  relop===  const=CollisionSeverity.None  OutDataTypeStr=boolean
  Compare To Constant1 : simulink/Logic and Bit Operations/Compare To Constant  relop=>=  const=P_AEB_Func_pedPrewarningMinActivationTimeAborThresSeverity  OutDataTypeStr=boolean
  Logical Operator : Logic  AllPortsSameDT=off  OutDataTypeStr=boolean
  pedPreWarningSituationRelAborCondFulfilled : Outport
  wires:
  Compare To Constant1/1 -> Logical Operator/2
  pedPreWarningWorkTime/1 -> Compare To Constant1/1
  Compare To Constant/1 -> Logical Operator/1
  latCollisionSeverity/1 -> Compare To Constant/1
  Logical Operator/1 -> pedPreWarningSituationRelAborCondFulfilled/1

== system TosAeb/FcwAebJa_Step_sys/ALG_AEBSystem/ALG_AEBController/AEB_FuncCondCalcApp/PedFunctionCondCalc/pedPreWarningSuppAborCond/pedPreWarningAborCondCalc/pedPreWarningVehRelAborCondCalc ==
  note: 取消速度限制
  vehStateCalcPreWarningAborFlg : Inport
  pedPreWarningWorkTime : Inport  Port=2
  Compare To Constant2 : simulink/Logic and Bit Operations/Compare To Constant  relop=>=  const=P_AEB_Func_pedPrewarningMaxActivationTimeAborThres  OutDataTypeStr=boolean
  Compare To Constant3 : simulink/Logic and Bit Operations/Compare To Constant  relop===  const=TRUE  OutDataTypeStr=boolean
  Compare To Constant4 : simulink/Logic and Bit Operations/Compare To Constant  relop=>=  const=P_AEB_Func_pedPrewarningMinActivationTimeAborThresVSC  OutDataTypeStr=boolean
  Logical Operator1 : Logic  AllPortsSameDT=off  OutDataTypeStr=boolean
  Logical Operator2 : Logic  Operator=OR  AllPortsSameDT=off  OutDataTypeStr=boolean
  pedPreWarningVehRelAborCondFulfilled : Outport
  wires:
  Logical Operator2/1 -> pedPreWarningVehRelAborCondFulfilled/1
  vehStateCalcPreWarningAborFlg/1 -> Compare To Constant3/1
  pedPreWarningWorkTime/1 -> Compare To Constant4/1, Compare To Constant2/1
  Compare To Constant2/1 -> Logical Operator2/2  "pedPreWarningWorkTimeRelCondFulfilled"
  Logical Operator1/1 -> Logical Operator2/1  "vehStateCalcPrefillAborCondFulfilled"
  Compare To Constant4/1 -> Logical Operator1/2
  Compare To Constant3/1 -> Logical Operator1/1

== system TosAeb/FcwAebJa_Step_sys/ALG_AEBSystem/ALG_AEBController/AEB_FuncCondCalcApp/PedFunctionCondCalc/pedPreWarningSuppAborCond/pedPreWarningSuppCondCalc/pedPreWarningObjRelActiveCondCalc ==
  ObjectID : Inport
  vxAbsObj : Inport  Port=2
  ObjFusQuality : Inport  Port=3
  dy : Inport  Port=4
  vy : Inport  Port=5
  Abs : Abs
  Compare To Constant : simulink/Logic and Bit Operations/Compare To Constant  relop=>  const=CNNull  OutDataTypeStr=boolean
  Compare To Constant1 : simulink/Logic and Bit Operations/Compare To Constant  relop=>  const=P_AEB_Func_pedPreWarningVxAbsObjSuppThres  OutDataTypeStr=boolean
  Compare To Constant2 : simulink/Logic and Bit Operations/Compare To Constant  relop===  const=FALSE  OutDataTypeStr=boolean
  Compare To Constant3 : simulink/Logic and Bit Operations/Compare To Constant  relop=>  const=CNNull  OutDataTypeStr=boolean
  Compare To Constant4 : simulink/Logic and Bit Operations/Compare To Constant  relop===  const=CNobjInvalidID  OutDataTypeStr=boolean
  Compare To Constant5 : simulink/Logic and Bit Operations/Compare To Constant  relop=<  const=CNNull  OutDataTypeStr=boolean
  Compare To Constant6 : simulink/Logic and Bit Operations/Compare To Constant  relop=<  const=CNNull  OutDataTypeStr=boolean
  Logical Operator : Logic  Operator=OR  Inputs=3  AllPortsSameDT=off  OutDataTypeStr=boolean
  Logical Operator1 : Logic  AllPortsSameDT=off  OutDataTypeStr=boolean
  Logical Operator2 : Logic  AllPortsSameDT=off  OutDataTypeStr=boolean
  Logical Operator3 : Logic  Operator=OR  AllPortsSameDT=off  OutDataTypeStr=boolean
  Terminator : Terminator
  pedPreWarningObjRelSuppCondFulfilled : Outport
  wires:
  Compare To Constant2/1 -> Logical Operator/3  "ObjFusQuality"
  ObjFusQuality/1 -> Compare To Constant2/1
  vy/1 -> Compare To Constant3/1, Compare To Constant6/1
  Compare To Constant3/1 -> Logical Operator1/2
  dy/1 -> Compare To Constant/1, Compare To Constant5/1
  Logical Operator2/1 -> Logical Operator3/2
  Compare To Constant/1 -> Logical Operator1/1
  Compare To Constant5/1 -> Logical Operator2/1
  Logical Operator1/1 -> Logical Operator3/1
  Compare To Constant6/1 -> Logical Operator2/2
  Abs/1 -> Compare To Constant1/1
  vxAbsObj/1 -> Abs/1
  Compare To Constant1/1 -> Logical Operator/2
  Logical Operator/1 -> pedPreWarningObjRelSuppCondFulfilled/1
  Compare To Constant4/1 -> Logical Operator/1
  ObjectID/1 -> Compare To Constant4/1
  Logical Operator3/1 -> Terminator/1

== system TosAeb/FcwAebJa_Step_sys/ALG_AEBSystem/ALG_AEBController/AEB_FuncCondCalcApp/PedFunctionCondCalc/pedPreWarningSuppAborCond/pedPreWarningSuppCondCalc/pedPreWarningSituationRelActiveCondCalc ==
  latCollisionSeverity : Inport
  subjCurvTraj : Inport  Port=2
  subjLatAccel : Inport  Port=3
  ObjectTypeCameraConfirmed : Inport  Port=4
  colliisonRiskProb : Inport  Port=5
  vy : Inport  Port=6
  dxFrontBumper : Inport  Port=7
  FcwHMISetting : Inport  Port=8
  subjVel : Inport  Port=9
  PreWarningMaxTimeCalc : Subsystem
  longDistMinCond4PreWarningCalc : Subsystem
  pedPreWarningSituationRelSuppCond : Subsystem
  vyCorrCalc : Subsystem
  pedPreWarningSituationRelSuppCondFulfilled : Outport
  wires:
  FcwHMISetting/1 -> PreWarningMaxTimeCalc/1(FcwHMISetting)
  subjVel/1 -> longDistMinCond4PreWarningCalc/2(subjVel), PreWarningMaxTimeCalc/2(subjVel)
  vyCorrCalc/1(objLatVyCond4Prewarning) -> pedPreWarningSituationRelSuppCond/6(pedLatVyCond4Prewarning)
  longDistMinCond4PreWarningCalc/1(longDistMinCond4PreWarning) -> pedPreWarningSituationRelSuppCond/8(longDistMinCond4PreWarning)
  PreWarningMaxTimeCalc/1(longDistCond4PreWarning) -> pedPreWarningSituationRelSuppCond/7(longDistCond4PreWarning)
  pedPreWarningSituationRelSuppCond/1(pedPreWarningSituationRelSuppCondFulfilled) -> pedPreWarningSituationRelSuppCondFulfilled/1
  ObjectTypeCameraConfirmed/1 -> pedPreWarningSituationRelSuppCond/5(ObjectTypeCameraConfirmed), vyCorrCalc/1(ObjectTypeCameraConfirmed)
  colliisonRiskProb/1 -> pedPreWarningSituationRelSuppCond/4(colliisonRiskProb)
  subjLatAccel/1 -> pedPreWarningSituationRelSuppCond/3(subjLatAccel)
  subjCurvTraj/1 -> pedPreWarningSituationRelSuppCond/2(subjCurvTraj)
  latCollisionSeverity/1 -> pedPreWarningSituationRelSuppCond/1(latCollisionSeverity)
  vy/1 -> vyCorrCalc/2(vy)
  dxFrontBumper/1 -> PreWarningMaxTimeCalc/3(dxFrontBumper), longDistMinCond4PreWarningCalc/1(dxFrontBumper)  "dxFrontBumper"

== system TosAeb/FcwAebJa_Step_sys/ALG_AEBSystem/ALG_AEBController/AEB_FuncCondCalcApp/PedFunctionCondCalc/pedPreWarningSuppAborCond/pedPreWarningSuppCondCalc/pedPreWarningSuppCondFinalJudge ==
  pedPreWarningObjRelSuppCondFulfilled : Inport
  pedPreWarningVehRelSuppCondFulfilled : Inport  Port=2
  pedPreWarningSituationRelSuppCondFulfilled : Inport  Port=3
  Compare To Constant : simulink/Logic and Bit Operations/Compare To Constant  relop===  const=TRUE  OutDataTypeStr=boolean
  Compare To Constant1 : simulink/Logic and Bit Operations/Compare To Constant  relop===  const=TRUE  OutDataTypeStr=boolean
  Compare To Constant2 : simulink/Logic and Bit Operations/Compare To Constant  relop===  const=TRUE  OutDataTypeStr=boolean
  Logical Operator : Logic  Operator=OR  Inputs=3  AllPortsSameDT=off  OutDataTypeStr=boolean
  pedPreWarningSuppCondFulfilled : Outport
  wires:
  Compare To Constant2/1 -> Logical Operator/3
  Compare To Constant1/1 -> Logical Operator/2
  Compare To Constant/1 -> Logical Operator/1
  pedPreWarningVehRelSuppCondFulfilled/1 -> Compare To Constant1/1
  pedPreWarningObjRelSuppCondFulfilled/1 -> Compare To Constant/1
  pedPreWarningSituationRelSuppCondFulfilled/1 -> Compare To Constant2/1
  Logical Operator/1 -> pedPreWarningSuppCondFulfilled/1

== system TosAeb/FcwAebJa_Step_sys/ALG_AEBSystem/ALG_AEBController/AEB_FuncCondCalcApp/PedFunctionCondCalc/pedPreWarningSuppAborCond/pedPreWarningSuppCondCalc/pedPreWarningVehRelActiveCondCalc ==
  subjVel : Inport
  VehConfigIbooster : Inport  Port=2
  ESP_MasterCylindBrakePressValid : Inport  Port=3
  ESP_MasterCylindBrakePress : Inport  Port=4
  IB_BrkPedalAppld : Inport  Port=5
  SteerWheelAngle : Inport  Port=6
  SteerWheelSpd : Inport  Port=7
  AccrPedlPosnDiagc : Inport  Port=8
  vehStateCalcPedPreWarningSuppFlg : Inport  Port=9
  Abs : Abs
  Abs1 : Abs
  AccReleventSuppCondDelayAbortion : AEBSFunctionLib/StateDelayAbortion  SystemSampleTime=-1  VariantActivationTime=update diagram  Latency=0  AutoFrameSizeCalculation=off
  Compare To Constant : simulink/Logic and Bit Operations/Compare To Constant  relop=>  const=P_AEB_Func_pedPrewarningStrWheelAngleSupThres  OutDataTypeStr=boolean
  Compare To Constant1 : simulink/Logic and Bit Operations/Compare To Constant  relop=>  const=P_AEB_Func_pedPrewarningGasPedalSupThres  OutDataTypeStr=boolean
  Compare To Constant2 : simulink/Logic and Bit Operations/Compare To Constant  relop=>  const=P_AEB_Func_pedPrewarningStrWheelAngleSpdSupThres  OutDataTypeStr=boolean
  Compare To Constant7 : simulink/Logic and Bit Operations/Compare To Constant  relop===  const=TRUE  OutDataTypeStr=boolean
  Compare To Constant8 : simulink/Logic and Bit Operations/Compare To Constant  relop=>  const=P_AEB_Func_pedPrewarningSubjVelMax  OutDataTypeStr=boolean
  Compare To Constant9 : simulink/Logic and Bit Operations/Compare To Constant  relop=<  const=P_AEB_Func_pedPrewarningSubjVelMin  OutDataTypeStr=boolean
  Constant : Constant  Value=P_AEB_Func_pedPrewarningStrCondAborDelayTime
  Constant1 : Constant  Value=0.02
  Constant2 : Constant  Value=P_AEB_Func_pedPrewarningAccrCondAborDelayTime
  Constant3 : Constant  Value=0.02
  Logical Operator : Logic  Operator=OR  AllPortsSameDT=off  OutDataTypeStr=boolean
  Logical Operator4 : Logic  Operator=OR  AllPortsSameDT=off  OutDataTypeStr=boolean
  Logical Operator5 : Logic  Operator=OR  Inputs=5  AllPortsSameDT=off  OutDataTypeStr=boolean
  StrReleventSuppCondDelayAbortion : AEBSFunctionLib/StateDelayAbortion  SystemSampleTime=-1  VariantActivationTime=update diagram  Latency=0  AutoFrameSizeCalculation=off
  brakeReleventSuppCond : Subsystem
  pedPreWarningVehRelSuppCondFulfilled : Outport
  wires:
  VehConfigIbooster/1 -> brakeReleventSuppCond/1(VehConfigIbooster)
  ESP_MasterCylindBrakePressValid/1 -> brakeReleventSuppCond/2(ESP_MasterCylindBrakePressValid)
  ESP_MasterCylindBrakePress/1 -> brakeReleventSuppCond/3(ESP_MasterCylindBrakePress)
  IB_BrkPedalAppld/1 -> brakeReleventSuppCond/4(IB_BrkPedalAppld)
  Constant2/1 -> AccReleventSuppCondDelayAbortion/2
  Constant3/1 -> AccReleventSuppCondDelayAbortion/3
  Compare To Constant1/1 -> AccReleventSuppCondDelayAbortion/1
  Logical Operator4/1 -> StrReleventSuppCondDelayAbortion/1
  Constant1/1 -> StrReleventSuppCondDelayAbortion/3
  Constant/1 -> StrReleventSuppCondDelayAbortion/2
  AccReleventSuppCondDelayAbortion/1 -> Logical Operator5/4  "accReleventSuppCondFulfilled"
  Abs1/1 -> Compare To Constant2/1
  vehStateCalcPedPreWarningSuppFlg/1 -> Compare To Constant7/1
  Compare To Constant8/1 -> Logical Operator/1
  Compare To Constant2/1 -> Logical Operator4/2
  SteerWheelSpd/1 -> Abs1/1
  Compare To Constant/1 -> Logical Operator4/1
  Abs/1 -> Compare To Constant/1
  SteerWheelAngle/1 -> Abs/1
  StrReleventSuppCondDelayAbortion/1 -> Logical Operator5/3  "strReleventSuppCondFulfilled"
  brakeReleventSuppCond/1(brakeReleventSuppCond) -> Logical Operator5/2  "BrkReleventSuppCondFulfilled"
  Compare To Constant9/1 -> Logical Operator/2
  AccrPedlPosnDiagc/1 -> Compare To Constant1/1
  Compare To Constant7/1 -> Logical Operator5/5  "vehStateCalcPedPrefillSuppCondFulfilled"
  Logical Operator/1 -> Logical Operator5/1  "subjVelReleventSuppCondFulfilled"
  subjVel/1 -> Compare To Constant9/1, Compare To Constant8/1
  Logical Operator5/1 -> pedPreWarningVehRelSuppCondFulfilled/1  "pedPrefillVehRelSuppCondFulfilled"

== system TosAeb/FcwAebJa_Step_sys/ALG_AEBSystem/ALG_AEBController/AEB_FuncCondCalcApp/PedFunctionCondCalc/pedPrefillSuppAborCond/pedPrefillAborCondCalc/pedPrefillAborCondFinalJudge ==
  pedPrefillObjRelAborCondFulfilled : Inport
  pedPrefillVehRelAborCondFulfilled : Inport  Port=2
  pedPrefillSituationRelAborCondFulfilled : Inport  Port=3
  Compare To Constant1 : simulink/Logic and Bit Operations/Compare To Constant  relop===  const=TRUE  OutDataTypeStr=boolean
  Compare To Constant2 : simulink/Logic and Bit Operations/Compare To Constant  relop===  const=TRUE  OutDataTypeStr=boolean
  Compare To Constant3 : simulink/Logic and Bit Operations/Compare To Constant  relop===  const=TRUE  OutDataTypeStr=boolean
  Logical Operator : Logic  Operator=OR  Inputs=3  AllPortsSameDT=off  OutDataTypeStr=boolean
  pedPrefillAborCondFulfilled : Outport
  wires:
  Compare To Constant3/1 -> Logical Operator/1
  pedPrefillVehRelAborCondFulfilled/1 -> Compare To Constant1/1
  pedPrefillSituationRelAborCondFulfilled/1 -> Compare To Constant2/1
  Logical Operator/1 -> pedPrefillAborCondFulfilled/1  "vehPreWarningAborCondFulfilled"
  pedPrefillObjRelAborCondFulfilled/1 -> Compare To Constant3/1
  Compare To Constant1/1 -> Logical Operator/2
  Compare To Constant2/1 -> Logical Operator/3

== system TosAeb/FcwAebJa_Step_sys/ALG_AEBSystem/ALG_AEBController/AEB_FuncCondCalcApp/PedFunctionCondCalc/pedPrefillSuppAborCond/pedPrefillAborCondCalc/pedPrefillObjRelAborCondCalc ==
  ObjectID : Inport
  Compare To Constant4 : simulink/Logic and Bit Operations/Compare To Constant  relop===  const=CNobjInvalidID  OutDataTypeStr=boolean
  pedPrefillObjRelAborCondFulfilled : Outport
  wires:
  Compare To Constant4/1 -> pedPrefillObjRelAborCondFulfilled/1
  ObjectID/1 -> Compare To Constant4/1

== system TosAeb/FcwAebJa_Step_sys/ALG_AEBSystem/ALG_AEBController/AEB_FuncCondCalcApp/PedFunctionCondCalc/pedPrefillSuppAborCond/pedPrefillAborCondCalc/pedPrefillSituationRelAborCondCalc ==
  note: 更改数值
  PedCollisionSeverity : Inport
  pedPrefillWorkTime : Inport  Port=2
  Compare To Constant : simulink/Logic and Bit Operations/Compare To Constant  relop===  const=CollisionSeverity.None  OutDataTypeStr=boolean
  Compare To Constant1 : simulink/Logic and Bit Operations/Compare To Constant  relop=>=  const=P_AEB_Func_pedPrefillMinActivationTimeAborThres  OutDataTypeStr=boolean
  Logical Operator : Logic  AllPortsSameDT=off  OutDataTypeStr=boolean
  pedPrefillSituationRelAborCondFulfilled : Outport
  wires:
  Compare To Constant1/1 -> Logical Operator/2
  pedPrefillWorkTime/1 -> Compare To Constant1/1
  Logical Operator/1 -> pedPrefillSituationRelAborCondFulfilled/1
  Compare To Constant/1 -> Logical Operator/1
  PedCollisionSeverity/1 -> Compare To Constant/1

== system TosAeb/FcwAebJa_Step_sys/ALG_AEBSystem/ALG_AEBController/AEB_FuncCondCalcApp/PedFunctionCondCalc/pedPrefillSuppAborCond/pedPrefillAborCondCalc/pedPrefillVehRelAborCondCalc ==
  SteerWheelAngle : Inport
  SteerWheelSpd : Inport  Port=2
  vehStateCalcPedPrefillAborFlg : Inport  Port=3
  pedPrefillWorkTime : Inport  Port=4
  Abs : Abs
  Abs1 : Abs
  Compare To Constant : simulink/Logic and Bit Operations/Compare To Constant  relop=>  const=P_AEB_Func_pedPrefillStrWheelAngleAborThres  OutDataTypeStr=boolean
  Compare To Constant1 : simulink/Logic and Bit Operations/Compare To Constant  relop=>  const=P_AEB_Func_pedPrefillStrWheelAngleSpdAborThres  OutDataTypeStr=boolean
  Compare To Constant2 : simulink/Logic and Bit Operations/Compare To Constant  relop=>  const=P_AEB_Func_pedPrefillMaxActivationTimeAborThres  OutDataTypeStr=boolean
  Compare To Constant3 : simulink/Logic and Bit Operations/Compare To Constant  relop===  const=TRUE  OutDataTypeStr=boolean
  Logical Operator : Logic  Operator=OR  AllPortsSameDT=off  OutDataTypeStr=boolean
  Logical Operator2 : Logic  Operator=OR  Inputs=3  AllPortsSameDT=off  OutDataTypeStr=boolean
  pedPrefillVehRelAborCondFulfilled : Outport
  wires:
  Compare To Constant2/1 -> Logical Operator2/3  "pedPrefillWorkTimeRelCondFulfilled"
  Logical Operator2/1 -> pedPrefillVehRelAborCondFulfilled/1
  Logical Operator/1 -> Logical Operator2/1  "strReleventAborCondFulfilled"
  Compare To Constant/1 -> Logical Operator/1
  vehStateCalcPedPrefillAborFlg/1 -> Compare To Constant3/1
  pedPrefillWorkTime/1 -> Compare To Constant2/1
  SteerWheelAngle/1 -> Abs/1
  Compare To Constant1/1 -> Logical Operator/2
  Abs1/1 -> Compare To Constant1/1
  SteerWheelSpd/1 -> Abs1/1
  Abs/1 -> Compare To Constant/1
  Compare To Constant3/1 -> Logical Operator2/2  "vehStateCalcPrefillAborCondFulfilled"
